simulink model "SdbRxSigProc"  (R2022b) (continued)
== system SdbRxSigProc == (continued)
  SdbRxSigProc_Runnable_sys/64(HmiClimaRow3PwrNgcc_HmiClimaRow3PwrNgcc_write) -> HmiClimaRow3PwrNgcc_HmiClimaRow3PwrNgcc/1
  SdbRxSigProc_Runnable_sys/65(HMIClimaVentSetNgcc_HMIClimaVentSetNgcc_write) -> HMIClimaVentSetNgcc_HMIClimaVentSetNgcc/1
  SdbRxSigProc_Runnable_sys/66(HmiDrvrHeadPosnNgcc_HmiDrvrHeadPosnNgcc_write) -> HmiDrvrHeadPosnNgcc_HmiDrvrHeadPosnNgcc/1
  SdbRxSigProc_Runnable_sys/67(HmiElecAirBodyVentLebtnNgcc_HmiElecAirBodyVentLebtnNgcc_write) -> HmiElecAirBodyVentLebtnNgcc_HmiElecAirBodyVentLebtnNgcc/1
  SdbRxSigProc_Runnable_sys/68(HmiElecAirBodyVentRibtnNgcc_HmiElecAirBodyVentRibtnNgcc_write) -> HmiElecAirBodyVentRibtnNgcc_HmiElecAirBodyVentRibtnNgcc/1
  SdbRxSigProc_Runnable_sys/69(HmiElecAirConsolePosnNgcc_HmiElecAirConsolePosnNgcc_write) -> HmiElecAirConsolePosnNgcc_HmiElecAirConsolePosnNgcc/1
  SdbRxSigProc_Runnable_sys/70(HmiElecAirConsoleSwtLeNgcc_HmiElecAirConsoleSwtLeNgcc_write) -> HmiElecAirConsoleSwtLeNgcc_HmiElecAirConsoleSwtLeNgcc/1
  SdbRxSigProc_Runnable_sys/71(HmiElecAirConsoleSwtRiNgcc_HmiElecAirConsoleSwtRiNgcc_write) -> HmiElecAirConsoleSwtRiNgcc_HmiElecAirConsoleSwtRiNgcc/1
  SdbRxSigProc_Runnable_sys/72(HmiElecAirDirModeSecRowReqNgcc_HmiElecAirDirModeSecRowReqNgcc_write) -> HmiElecAirDirModeSecRowReqNgcc_HmiElecAirDirModeSecRowReqNgcc/1
  SdbRxSigProc_Runnable_sys/73(HmiElecAirDirSwngSecLeNgcc_HmiElecAirDirSwngSecLeNgcc_write) -> HmiElecAirDirSwngSecLeNgcc_HmiElecAirDirSwngSecLeNgcc/1
  SdbRxSigProc_Runnable_sys/74(HmiElecAirDirSwngSecRiNgcc_HmiElecAirDirSwngSecRiNgcc_write) -> HmiElecAirDirSwngSecRiNgcc_HmiElecAirDirSwngSecRiNgcc/1
  SdbRxSigProc_Runnable_sys/75(HmiElecAirDrvrNatureWindReqNgcc_HmiElecAirDrvrNatureWindReqNgcc_write) -> HmiElecAirDrvrNatureWindReqNgcc_HmiElecAirDrvrNatureWindReqNgcc/1
  SdbRxSigProc_Runnable_sys/76(HmiElecAirPassNatureWindReqNgcc_HmiElecAirPassNatureWindReqNgcc_write) -> HmiElecAirPassNatureWindReqNgcc_HmiElecAirPassNatureWindReqNgcc/1
  SdbRxSigProc_Runnable_sys/77(HMIFACSwtNgcc_HMIFACSwtNgcc_write) -> HMIFACSwtNgcc_HMIFACSwtNgcc/1
  SdbRxSigProc_Runnable_sys/78(HmiFanLvlRow1LeNgcc_HmiFanLvlRow1LeNgcc_write) -> HmiFanLvlRow1LeNgcc_HmiFanLvlRow1LeNgcc/1
  SdbRxSigProc_Runnable_sys/79(HmiFanLvlRow1RiNgcc_HmiFanLvlRow1RiNgcc_write) -> HmiFanLvlRow1RiNgcc_HmiFanLvlRow1RiNgcc/1
  SdbRxSigProc_Runnable_sys/80(HmiFragraChRatReq_HmiFragraChRatReq_write) -> HmiFragraChRatReq_HmiFragraChRatReq/1
  SdbRxSigProc_Runnable_sys/81(HmiFragraLvlReq_HmiFragraLvlReq_write) -> HmiFragraLvlReq_HmiFragraLvlReq/1
  SdbRxSigProc_Runnable_sys/82(HMILBRACStrtSetNgcc_HMILBRACStrtSetNgcc_write) -> HMILBRACStrtSetNgcc_HMILBRACStrtSetNgcc/1
  SdbRxSigProc_Runnable_sys/83(HmiOxyinCarSwtNgcc_HmiOxyinCarSwtNgcc_write) -> HmiOxyinCarSwtNgcc_HmiOxyinCarSwtNgcc/1
  SdbRxSigProc_Runnable_sys/84(HmiParkVentSetNgcc_HmiParkVentSetNgcc_write) -> HmiParkVentSetNgcc_HmiParkVentSetNgcc/1
  SdbRxSigProc_Runnable_sys/85(HmiParkVentTmrSetNgcc_HmiParkVentTmrSetNgcc_write) -> HmiParkVentTmrSetNgcc_HmiParkVentTmrSetNgcc/1
  SdbRxSigProc_Runnable_sys/86(HmiPassHeadPosnNgcc_HmiPassHeadPosnNgcc_write) -> HmiPassHeadPosnNgcc_HmiPassHeadPosnNgcc/1
  SdbRxSigProc_Runnable_sys/87(HmiPopUpResp_HmiPopUpResp_write) -> HmiPopUpResp_HmiPopUpResp/1
  SdbRxSigProc_Runnable_sys/88(HmiSmrtWindFolwSetNgcc_HmiSmrtWindFolwSetNgcc_write) -> HmiSmrtWindFolwSetNgcc_HmiSmrtWindFolwSetNgcc/1
  SdbRxSigProc_Runnable_sys/89(HMIThermoTankDoorOpenNgcc_HMIThermoTankDoorOpenNgcc_write) -> HMIThermoTankDoorOpenNgcc_HMIThermoTankDoorOpenNgcc/1
  SdbRxSigProc_Runnable_sys/90(HMIThermoTankEcoSwNgcc_HMIThermoTankEcoSwNgcc_write) -> HMIThermoTankEcoSwNgcc_HMIThermoTankEcoSwNgcc/1
  SdbRxSigProc_Runnable_sys/91(HMIThermoTankTNgcc_HMIThermoTankTNgcc_write) -> HMIThermoTankTNgcc_HMIThermoTankTNgcc/1
  SdbRxSigProc_Runnable_sys/92(HmiThermoTSteDeoNewSwtNgcc_HmiThermoTSteDeoNewSwtNgcc_write) -> HmiThermoTSteDeoNewSwtNgcc_HmiThermoTSteDeoNewSwtNgcc/1
  SdbRxSigProc_Runnable_sys/93(HmiThermoTSteDeoSwtNgcc_HmiThermoTSteDeoSwtNgcc_write) -> HmiThermoTSteDeoSwtNgcc_HmiThermoTSteDeoSwtNgcc/1
  SdbRxSigProc_Runnable_sys/94(HmiUVSterilSetNgcc_HmiUVSterilSetNgcc_write) -> HmiUVSterilSetNgcc_HmiUVSterilSetNgcc/1
  SdbRxSigProc_Runnable_sys/95(HmiWinterLockCarPrevnIceSetNgcc_HmiWinterLockCarPrevnIceSetNgcc_write) -> HmiWinterLockCarPrevnIceSetNgcc_HmiWinterLockCarPrevnIceSetNgcc/1
  SdbRxSigProc_Runnable_sys/96(HoodStsNgcc_HoodStsNgcc_write) -> HoodStsNgcc_HoodStsNgcc/1
  SdbRxSigProc_Runnable_sys/97(HvBattLimnIndcnNgcc_HvBattLimnIndcnNgcc_write) -> HvBattLimnIndcnNgcc_HvBattLimnIndcnNgcc/1
  SdbRxSigProc_Runnable_sys/98(InflateAirSrcSwtNgcc_InflateAirSrcSwtNgcc_write) -> InflateAirSrcSwtNgcc_InflateAirSrcSwtNgcc/1
  SdbRxSigProc_Runnable_sys/99(InpVoltFbNgcc_InpVoltFbNgcc_write) -> InpVoltFbNgcc_InpVoltFbNgcc/1
  SdbRxSigProc_Runnable_sys/100(LiOprnModNgcc_LiOprnModNgcc_write) -> LiOprnModNgcc_LiOprnModNgcc/1
  SdbRxSigProc_Runnable_sys/101(LonglifeBatteryReqNgcc_LonglifeBatteryReqNgcc_write) -> LonglifeBatteryReqNgcc_LonglifeBatteryReqNgcc/1
  SdbRxSigProc_Runnable_sys/102(McuOvrTempErrNgcc_McuOvrTempErrNgcc_write) -> McuOvrTempErrNgcc_McuOvrTempErrNgcc/1
  SdbRxSigProc_Runnable_sys/103(McuTempFbNgcc_McuTempFbNgcc_write) -> McuTempFbNgcc_McuTempFbNgcc/1
  SdbRxSigProc_Runnable_sys/104(McuTempSnsrErrNgcc_McuTempSnsrErrNgcc_write) -> McuTempSnsrErrNgcc_McuTempSnsrErrNgcc/1
  SdbRxSigProc_Runnable_sys/105(MoleculeVlv1Ngcc_MoleculeVlv1Ngcc_write) -> MoleculeVlv1Ngcc_MoleculeVlv1Ngcc/1
  SdbRxSigProc_Runnable_sys/106(MoleculeVlv2Ngcc_MoleculeVlv2Ngcc_write) -> MoleculeVlv2Ngcc_MoleculeVlv2Ngcc/1
  SdbRxSigProc_Runnable_sys/107(MosOvrTempErrNgcc_MosOvrTempErrNgcc_write) -> MosOvrTempErrNgcc_MosOvrTempErrNgcc/1
  SdbRxSigProc_Runnable_sys/108(MosTempFbNgcc_MosTempFbNgcc_write) -> MosTempFbNgcc_MosTempFbNgcc/1
  SdbRxSigProc_Runnable_sys/109(MosTempSnsrErrNgcc_MosTempSnsrErrNgcc_write) -> MosTempSnsrErrNgcc_MosTempSnsrErrNgcc/1
  SdbRxSigProc_Runnable_sys/110(MotStsFrmOGMNgcc_MotStsFrmOGMNgcc_write) -> MotStsFrmOGMNgcc_MotStsFrmOGMNgcc/1
  SdbRxSigProc_Runnable_sys/111(MotTempFbNgcc_MotTempFbNgcc_write) -> MotTempFbNgcc_MotTempFbNgcc/1
  SdbRxSigProc_Runnable_sys/112(MotTempSnsrErrNgcc_MotTempSnsrErrNgcc_write) -> MotTempSnsrErrNgcc_MotTempSnsrErrNgcc/1
  SdbRxSigProc_Runnable_sys/113(OGMCmprSpdFbNgcc_OGMCmprSpdFbNgcc_write) -> OGMCmprSpdFbNgcc_OGMCmprSpdFbNgcc/1
  SdbRxSigProc_Runnable_sys/114(OGMFlowFbNgcc_OGMFlowFbNgcc_write) -> OGMFlowFbNgcc_OGMFlowFbNgcc/1
  SdbRxSigProc_Runnable_sys/115(OGMHwVersNgcc_OGMHwVersNgcc_write) -> OGMHwVersNgcc_OGMHwVersNgcc/1
  SdbRxSigProc_Runnable_sys/116(OGMIntErrNgcc_OGMIntErrNgcc_write) -> OGMIntErrNgcc_OGMIntErrNgcc/1
  SdbRxSigProc_Runnable_sys/117(OGMOvrVoltRangErrNgcc_OGMOvrVoltRangErrNgcc_write) -> OGMOvrVoltRangErrNgcc_OGMOvrVoltRangErrNgcc/1
  SdbRxSigProc_Runnable_sys/118(OGMRunTiNgcc_OGMRunTiNgcc_write) -> OGMRunTiNgcc_OGMRunTiNgcc/1
  SdbRxSigProc_Runnable_sys/119(OGMSwVersNgcc_OGMSwVersNgcc_write) -> OGMSwVersNgcc_OGMSwVersNgcc/1
  SdbRxSigProc_Runnable_sys/120(OGMTempErrNgcc_OGMTempErrNgcc_write) -> OGMTempErrNgcc_OGMTempErrNgcc/1
  SdbRxSigProc_Runnable_sys/121(OGMUndVoltRangErrNgcc_OGMUndVoltRangErrNgcc_write) -> OGMUndVoltRangErrNgcc_OGMUndVoltRangErrNgcc/1
  SdbRxSigProc_Runnable_sys/122(OxygenConcFbFrmOGMNgcc_OxygenConcFbFrmOGMNgcc_write) -> OxygenConcFbFrmOGMNgcc_OxygenConcFbFrmOGMNgcc/1
  SdbRxSigProc_Runnable_sys/123(OxygenConcFltNgcc_OxygenConcFltNgcc_write) -> OxygenConcFltNgcc_OxygenConcFltNgcc/1
  SdbRxSigProc_Runnable_sys/124(OxygenConcSnsrElecErrNgcc_OxygenConcSnsrElecErrNgcc_write) -> OxygenConcSnsrElecErrNgcc_OxygenConcSnsrElecErrNgcc/1
  SdbRxSigProc_Runnable_sys/125(OxygenConcSnsrIntErrNgcc_OxygenConcSnsrIntErrNgcc_write) -> OxygenConcSnsrIntErrNgcc_OxygenConcSnsrIntErrNgcc/1
  SdbRxSigProc_Runnable_sys/126(OxygenConcSnsrOvrVoltRangErrNgcc_OxygenConcSnsrOvrVoltRangErrNgcc_write) -> OxygenConcSnsrOvrVoltRangErrNgcc_OxygenConcSnsrOvrVoltRangErrNgcc/1
  SdbRxSigProc_Runnable_sys/127(OxygenConcSnsrStsFrmOGMNgcc_OxygenConcSnsrStsFrmOGMNgcc_write) -> OxygenConcSnsrStsFrmOGMNgcc_OxygenConcSnsrStsFrmOGMNgcc/1
  SdbRxSigProc_Runnable_sys/128(OxygenConcSnsrStsNgcc_OxygenConcSnsrStsNgcc_write) -> OxygenConcSnsrStsNgcc_OxygenConcSnsrStsNgcc/1
  SdbRxSigProc_Runnable_sys/129(OxygenConcSnsrTempErrNgcc_OxygenConcSnsrTempErrNgcc_write) -> OxygenConcSnsrTempErrNgcc_OxygenConcSnsrTempErrNgcc/1
  SdbRxSigProc_Runnable_sys/130(OxygenConcSnsrUndVoltRangErrNgcc_OxygenConcSnsrUndVoltRangErrNgcc_write) -> OxygenConcSnsrUndVoltRangErrNgcc_OxygenConcSnsrUndVoltRangErrNgcc/1
  SdbRxSigProc_Runnable_sys/131(OxygenGenrErrInfoNgcc_OxygenGenrErrInfoNgcc_write) -> OxygenGenrErrInfoNgcc_OxygenGenrErrInfoNgcc/1
  SdbRxSigProc_Runnable_sys/132(OxygenSplyAvlStsNgcc_OxygenSplyAvlStsNgcc_write) -> OxygenSplyAvlStsNgcc_OxygenSplyAvlStsNgcc/1
  SdbRxSigProc_Runnable_sys/133(OxygenSplyErrNgcc_OxygenSplyErrNgcc_write) -> OxygenSplyErrNgcc_OxygenSplyErrNgcc/1
  SdbRxSigProc_Runnable_sys/134(OxygenTubeBlkNgcc_OxygenTubeBlkNgcc_write) -> OxygenTubeBlkNgcc_OxygenTubeBlkNgcc/1
  SdbRxSigProc_Runnable_sys/135(PassSeatPosPercNgcc_PassSeatPosPercNgcc_write) -> PassSeatPosPercNgcc_PassSeatPosPercNgcc/1
  SdbRxSigProc_Runnable_sys/136(PassSeatSts2_Ngcc_PassSeatSts2_write) -> PassSeatSts2_Ngcc_PassSeatSts2/1
  SdbRxSigProc_Runnable_sys/137(PassSeatVentnLvlSts2Ngcc_PassSeatVentnLvlSts2Ngcc_write) -> PassSeatVentnLvlSts2Ngcc_PassSeatVentnLvlSts2Ngcc/1
  SdbRxSigProc_Runnable_sys/138(PhaseCurrentFbNgcc_PhaseCurrentFbNgcc_write) -> PhaseCurrentFbNgcc_PhaseCurrentFbNgcc/1
  SdbRxSigProc_Runnable_sys/139(PosnBriefNgcc_PosnBriefNgcc_write) -> PosnBriefNgcc_PosnBriefNgcc/1
  SdbRxSigProc_Runnable_sys/140(PressureFbNgcc_PressureFbNgcc_write) -> PressureFbNgcc_PressureFbNgcc/1
  SdbRxSigProc_Runnable_sys/141(PressureFltNgcc_PressureFltNgcc_write) -> PressureFltNgcc_PressureFltNgcc/1
  SdbRxSigProc_Runnable_sys/142(PressureSnsrErrNgcc_PressureSnsrErrNgcc_write) -> PressureSnsrErrNgcc_PressureSnsrErrNgcc/1
  SdbRxSigProc_Runnable_sys/143(PwrCnsForHvacHeatrElecRe_PwrCnsForHvacHeatrElecRe_write) -> PwrCnsForHvacHeatrElecRe_PwrCnsForHvacHeatrElecRe/1
  SdbRxSigProc_Runnable_sys/144(RefrLpSwStsNgcc_RefrLpSwStsNgcc_write) -> RefrLpSwStsNgcc_RefrLpSwStsNgcc/1
  SdbRxSigProc_Runnable_sys/145(RefrtorVlvEnaNgcc_RefrtorVlvEnaNgcc_write) -> RefrtorVlvEnaNgcc_RefrtorVlvEnaNgcc/1
  SdbRxSigProc_Runnable_sys/146(RoadInclnNgcc_RoadInclnNgcc_write) -> RoadInclnNgcc_RoadInclnNgcc/1
  SdbRxSigProc_Runnable_sys/147(RuranACModeSetNgcc_RuranACModeSetNgcc_write) -> RuranACModeSetNgcc_RuranACModeSetNgcc/1
  SdbRxSigProc_Runnable_sys/148(SceneModSeld_Ngcc_SceneModSeld_write) -> SceneModSeld_Ngcc_SceneModSeld/1
  SdbRxSigProc_Runnable_sys/149(SeatBackAngleRowFirstDrvrNgcc_SeatBackAngleRowFirstDrvrNgcc_write) -> SeatBackAngleRowFirstDrvrNgcc_SeatBackAngleRowFirstDrvrNgcc/1
  SdbRxSigProc_Runnable_sys/150(SeatBackAngleRowFirstPassNgcc_SeatBackAngleRowFirstPassNgcc_write) -> SeatBackAngleRowFirstPassNgcc_SeatBackAngleRowFirstPassNgcc/1
  SdbRxSigProc_Runnable_sys/151(SeatOccptAtRowSecDrSide2_Ngcc_SeatOccptAtRowSecDrSide2_write) -> SeatOccptAtRowSecDrSide2_Ngcc_SeatOccptAtRowSecDrSide2/1
  SdbRxSigProc_Runnable_sys/152(SeatOccptAtRowSecMid2_Ngcc_SeatOccptAtRowSecMid2_write) -> SeatOccptAtRowSecMid2_Ngcc_SeatOccptAtRowSecMid2/1
  SdbRxSigProc_Runnable_sys/153(SeatOccptAtRowSecPasSide2_Ngcc_SeatOccptAtRowSecPasSide2_write) -> SeatOccptAtRowSecPasSide2_Ngcc_SeatOccptAtRowSecPasSide2/1
  SdbRxSigProc_Runnable_sys/154(SeatOccptAtRowThrdLe2Ngcc_SeatOccptAtRowThrdLe2Ngcc_write) -> SeatOccptAtRowThrdLe2Ngcc_SeatOccptAtRowThrdLe2Ngcc/1
  SdbRxSigProc_Runnable_sys/155(SeatOccptAtRowThrdRi2Ngcc_SeatOccptAtRowThrdRi2Ngcc_write) -> SeatOccptAtRowThrdRi2Ngcc_SeatOccptAtRowThrdRi2Ngcc/1
  SdbRxSigProc_Runnable_sys/156(SportModeThmgtRequestNgcc_SportModeThmgtRequestNgcc_write) -> SportModeThmgtRequestNgcc_SportModeThmgtRequestNgcc/1
  SdbRxSigProc_Runnable_sys/157(TCSCooltFlowNgcc_TCSCooltFlowNgcc_write) -> TCSCooltFlowNgcc_TCSCooltFlowNgcc/1
  SdbRxSigProc_Runnable_sys/158(TCSCooltTNgcc_TCSCooltTNgcc_write) -> TCSCooltTNgcc_TCSCooltTNgcc/1
  SdbRxSigProc_Runnable_sys/159(TcsDoorEleBailReqNgcc_TcsDoorEleBailReqNgcc_write) -> TcsDoorEleBailReqNgcc_TcsDoorEleBailReqNgcc/1
  SdbRxSigProc_Runnable_sys/160(TcsFanReqNgcc_TcsFanReqNgcc_write) -> TcsFanReqNgcc_TcsFanReqNgcc/1
  SdbRxSigProc_Runnable_sys/161(TcsModSwtReqNgcc_TcsModSwtReqNgcc_write) -> TcsModSwtReqNgcc_TcsModSwtReqNgcc/1
  SdbRxSigProc_Runnable_sys/162(TcsOvrheatdProtnStsNgcc_TcsOvrheatdProtnStsNgcc_write) -> TcsOvrheatdProtnStsNgcc_TcsOvrheatdProtnStsNgcc/1
  SdbRxSigProc_Runnable_sys/163(TcsSemiconReqNgcc_TcsSemiconReqNgcc_write) -> TcsSemiconReqNgcc_TcsSemiconReqNgcc/1
  SdbRxSigProc_Runnable_sys/164(TcsTmrOverReqNgcc_TcsTmrOverReqNgcc_write) -> TcsTmrOverReqNgcc_TcsTmrOverReqNgcc/1
  SdbRxSigProc_Runnable_sys/165(TcsTNowCtrlReqNgcc_TcsTNowCtrlReqNgcc_write) -> TcsTNowCtrlReqNgcc_TcsTNowCtrlReqNgcc/1
  SdbRxSigProc_Runnable_sys/166(TcsTopDoorEleBailReqNgcc_TcsTopDoorEleBailReqNgcc_write) -> TcsTopDoorEleBailReqNgcc_TcsTopDoorEleBailReqNgcc/1
  SdbRxSigProc_Runnable_sys/167(TelmOxygenValReqNgcc_TelmOxygenValReqNgcc_write) -> TelmOxygenValReqNgcc_TelmOxygenValReqNgcc/1
  SdbRxSigProc_Runnable_sys/168(TelmThermoTankECOSetNgcc_TelmThermoTankECOSetNgcc_write) -> TelmThermoTankECOSetNgcc_TelmThermoTankECOSetNgcc/1
  SdbRxSigProc_Runnable_sys/169(TelmThermoTankTNgcc_TelmThermoTankTNgcc_write) -> TelmThermoTankTNgcc_TelmThermoTankTNgcc/1
  SdbRxSigProc_Runnable_sys/170(ThermHvacRecircReqEnaNgcc_ThermHvacRecircReqEnaNgcc_write) -> ThermHvacRecircReqEnaNgcc_ThermHvacRecircReqEnaNgcc/1
  SdbRxSigProc_Runnable_sys/171(ThermHvacRecircReqNgcc_ThermHvacRecircReqNgcc_write) -> ThermHvacRecircReqNgcc_ThermHvacRecircReqNgcc/1
  SdbRxSigProc_Runnable_sys/172(ThrdRowSeatHeatgActlPwrCnsLeNgcc_ThrdRowSeatHeatgActlPwrCnsLeNgcc_write) -> ThrdRowSeatHeatgActlPwrCnsLeNgcc_ThrdRowSeatHeatgActlPwrCnsLeNgcc/1
  SdbRxSigProc_Runnable_sys/173(ThrdRowSeatHeatgActlPwrCnsRiNgcc_ThrdRowSeatHeatgActlPwrCnsRiNgcc_write) -> ThrdRowSeatHeatgActlPwrCnsRiNgcc_ThrdRowSeatHeatgActlPwrCnsRiNgcc/1
  SdbRxSigProc_Runnable_sys/174(ThrdRowSeatHeatgDesPwrCnsLeNgcc_ThrdRowSeatHeatgDesPwrCnsLeNgcc_write) -> ThrdRowSeatHeatgDesPwrCnsLeNgcc_ThrdRowSeatHeatgDesPwrCnsLeNgcc/1
  SdbRxSigProc_Runnable_sys/175(ThrdRowSeatHeatgDesPwrCnsRiNgcc_ThrdRowSeatHeatgDesPwrCnsRiNgcc_write) -> ThrdRowSeatHeatgDesPwrCnsRiNgcc_ThrdRowSeatHeatgDesPwrCnsRiNgcc/1
  SdbRxSigProc_Runnable_sys/176(ThrdRowSeatVentnActlPwrCnsLeNgcc_ThrdRowSeatVentnActlPwrCnsLeNgcc_write) -> ThrdRowSeatVentnActlPwrCnsLeNgcc_ThrdRowSeatVentnActlPwrCnsLeNgcc/1
  SdbRxSigProc_Runnable_sys/177(ThrdRowSeatVentnActlPwrCnsRiNgcc_ThrdRowSeatVentnActlPwrCnsRiNgcc_write) -> ThrdRowSeatVentnActlPwrCnsRiNgcc_ThrdRowSeatVentnActlPwrCnsRiNgcc/1
  SdbRxSigProc_Runnable_sys/178(ThrdRowSeatVentnDesPwrCnsLeNgcc_ThrdRowSeatVentnDesPwrCnsLeNgcc_write) -> ThrdRowSeatVentnDesPwrCnsLeNgcc_ThrdRowSeatVentnDesPwrCnsLeNgcc/1
  SdbRxSigProc_Runnable_sys/179(ThrdRowSeatVentnDesPwrCnsRiNgcc_ThrdRowSeatVentnDesPwrCnsRiNgcc_write) -> ThrdRowSeatVentnDesPwrCnsRiNgcc_ThrdRowSeatVentnDesPwrCnsRiNgcc/1
  SdbRxSigProc_Runnable_sys/180(UseEvapReqTMinNewNgcc_UseEvapReqTMinNewNgcc_write) -> UseEvapReqTMinNewNgcc_UseEvapReqTMinNewNgcc/1
  SdbRxSigProc_Runnable_sys/181(VehTiAndDataNgcc_VehTiAndDataNgcc_write) -> VehTiAndDataNgcc_VehTiAndDataNgcc/1
  SdbRxSigProc_Runnable_sys/182(VlvVoltFltNgcc_VlvVoltFltNgcc_write) -> VlvVoltFltNgcc_VlvVoltFltNgcc/1
  SdbRxSigProc_Runnable_sys/183(WarnLvlMsgNgcc_WarnLvlMsgNgcc_write) -> WarnLvlMsgNgcc_WarnLvlMsgNgcc/1
  SdbRxSigProc_Runnable_sys/184(WasRespLevelNgcc_WasRespLevelNgcc_write) -> WasRespLevelNgcc_WasRespLevelNgcc/1
  SdbRxSigProc_Runnable_sys/185(WaterDepthInformationNgcc_WaterDepthInformationNgcc_write) -> WaterDepthInformationNgcc_WaterDepthInformationNgcc/1
  SdbRxSigProc_Runnable_sys/186(WeatherQualityAQINgcc_WeatherQualityAQINgcc_write) -> WeatherQualityAQINgcc_WeatherQualityAQINgcc/1
  SdbRxSigProc_Runnable_sys/187(WinPosnStsDrv_WinPosnStsAtDrv_write) -> WinPosnStsDrv_WinPosnStsAtDrv/1
  SdbRxSigProc_Runnable_sys/188(ActvnOfWshrFrntSafe_Ngcc_ActvnOfWshrFrntSafe_write) -> ActvnOfWshrFrntSafe_Ngcc_ActvnOfWshrFrntSafe/1
  SdbRxSigProc_Runnable_sys/189(AirMtrlSnsrInAirQlyIdx_AirMtrlSnsrInAirQlyIdx_write) -> AirMtrlSnsrInAirQlyIdx_AirMtrlSnsrInAirQlyIdx/1
  SdbRxSigProc_Runnable_sys/190(AirTAftHeatElmEstimd_AirTAftHeatElmEstimd_write) -> AirTAftHeatElmEstimd_AirTAftHeatElmEstimd/1
  SdbRxSigProc_Runnable_sys/191(AirTFromHeatgEstimd_Ngcc_AirTFromHeatgEstimd_write) -> AirTFromHeatgEstimd_Ngcc_AirTFromHeatgEstimd/1
  AmbTOutOfVeh_AmbTOutOfVeh_merge/1 -> AmbTOutOfVeh_AmbTOutOfVeh/1
  AmbTRaw_Ngcc_AmbTRaw_merge/1 -> AmbTRaw_Ngcc_AmbTRaw/1
  SdbRxSigProc_Runnable_sys/194(AsyFanSts_AsyFanSts_write) -> AsyFanSts_AsyFanSts/1
  SdbRxSigProc_Runnable_sys/195(BltLockStAtDrvr_Ngcc_BltLockStAtDrvr_write) -> BltLockStAtDrvr_Ngcc_BltLockStAtDrvr/1
  SdbRxSigProc_Runnable_sys/196(BltLockStAtPass_Ngcc_BltLockStAtPass_write) -> BltLockStAtPass_Ngcc_BltLockStAtPass/1
  SdbRxSigProc_Runnable_sys/197(BltLockStAtRowSecLe_Ngcc_BltLockStAtRowSecLe_write) -> BltLockStAtRowSecLe_Ngcc_BltLockStAtRowSecLe/1
  SdbRxSigProc_Runnable_sys/198(BltLockStAtRowSecMid_Ngcc_BltLockStAtRowSecMid_write) -> BltLockStAtRowSecMid_Ngcc_BltLockStAtRowSecMid/1
  SdbRxSigProc_Runnable_sys/199(BltLockStAtRowSecRi_Ngcc_BltLockStAtRowSecRi_write) -> BltLockStAtRowSecRi_Ngcc_BltLockStAtRowSecRi/1
  SdbRxSigProc_Runnable_sys/200(BltLockStAtRowThrdLe_Ngcc_BltLockStAtRowThrdLe_write) -> BltLockStAtRowThrdLe_Ngcc_BltLockStAtRowThrdLe/1
  SdbRxSigProc_Runnable_sys/201(BltLockStAtRowThrdRi_Ngcc_BltLockStAtRowThrdRi_write) -> BltLockStAtRowThrdRi_Ngcc_BltLockStAtRowThrdRi/1
  SdbRxSigProc_Runnable_sys/202(CamHeatrActv_CamHeatrActv_write) -> CamHeatrActv_CamHeatrActv/1
  SdbRxSigProc_Runnable_sys/203(CarTiGlb_Ngcc_CarTiGlb_write) -> CarTiGlb_Ngcc_CarTiGlb/1
  SdbRxSigProc_Runnable_sys/204(ClimaCmd_Ngcc_ClimaCmd_write) -> ClimaCmd_Ngcc_ClimaCmd/1
  SdbRxSigProc_Runnable_sys/205(ClimaPwrAllwd_Ngcc_ClimaPwrAllwd_write) -> ClimaPwrAllwd_Ngcc_ClimaPwrAllwd/1
  SdbRxSigProc_Runnable_sys/206(ClimaRqrd1ConnGen2_ClimaRqrd1ConnGen2_write) -> ClimaRqrd1ConnGen2_ClimaRqrd1ConnGen2/1
  SdbRxSigProc_Runnable_sys/207(ClimaRqrdFromRem1_ClimaRqrdFromRem1_write) -> ClimaRqrdFromRem1_ClimaRqrdFromRem1/1
  SdbRxSigProc_Runnable_sys/208(ClimaTmr_ClimaTmr_write) -> ClimaTmr_ClimaTmr/1
  SdbRxSigProc_Runnable_sys/209(ClimaTmrStsRqrd_ClimaTmrStsRqrd_write) -> ClimaTmrStsRqrd_ClimaTmrStsRqrd/1
  SdbRxSigProc_Runnable_sys/210(ClngRqrdFromHmi_ClngRqrdFromHmi_write) -> ClngRqrdFromHmi_ClngRqrdFromHmi/1
  SdbRxSigProc_Runnable_sys/211(ClngRqrdFromTelm_ClngRqrdFromTelm_write) -> ClngRqrdFromTelm_ClngRqrdFromTelm/1
  SdbRxSigProc_Runnable_sys/212(ClngRqrdFromTelmConnGen2_ClngRqrdFromTelmConnGen2_write) -> ClngRqrdFromTelmConnGen2_ClngRqrdFromTelmConnGen2/1
  SdbRxSigProc_Runnable_sys/213(CmpmtInAirPmAQI_Ngcc_CmpmtInAirPmAQI_write) -> CmpmtInAirPmAQI_Ngcc_CmpmtInAirPmAQI/1
  SdbRxSigProc_Runnable_sys/214(CmpmtInAirPmDnsty_Ngcc_CmpmtInAirPmDnsty_write) -> CmpmtInAirPmDnsty_Ngcc_CmpmtInAirPmDnsty/1
  SdbRxSigProc_Runnable_sys/215(CmptmtClngPreStrtReqNgcc_CmptmtClngPreStrtReq_write) -> CmptmtClngPreStrtReqNgcc_CmptmtClngPreStrtReq/1
  SdbRxSigProc_Runnable_sys/216(CmptmtCoolgSts_Ngcc_CmptmtCoolgSts_write) -> CmptmtCoolgSts_Ngcc_CmptmtCoolgSts/1
  CmptmtRelHum_Ngcc_CmptmtRelHum_merge/1 -> CmptmtRelHum_Ngcc_CmptmtRelHum/1
  SdbRxSigProc_Runnable_sys/218(CoolgLoad_CoolgLoad_write) -> CoolgLoad_CoolgLoad/1
  SdbRxSigProc_Runnable_sys/219(CoolgReqForDispCen_CoolgReqForDispCen_write) -> CoolgReqForDispCen_CoolgReqForDispCen/1
  SdbRxSigProc_Runnable_sys/220(CoolgReqForDrvrDisp_CoolgReqForDrvrDisp_write) -> CoolgReqForDrvrDisp_CoolgReqForDrvrDisp/1
  CooltHeatrAvlStsForPrkgClima_CooltHeatrAvlStsForPrkgClima_merge/1 -> CooltHeatrAvlStsForPrkgClima_CooltHeatrAvlStsForPrkgClima/1
  SdbRxSigProc_Runnable_sys/222(CooltTForPrkgClima_CooltTForPrkgClima_write) -> CooltTForPrkgClima_CooltTForPrkgClima/1
  SdbRxSigProc_Runnable_sys/223(CooltTInHeatElm_CooltTInHeatElm_write) -> CooltTInHeatElm_CooltTInHeatElm/1
  SdbRxSigProc_Runnable_sys/224(CooltVlvClsd_CooltVlvClsd_write) -> CooltVlvClsd_CooltVlvClsd/1
  SdbRxSigProc_Runnable_sys/225(CupHoldrDesPwr_CupHoldrDesPwr_write) -> CupHoldrDesPwr_CupHoldrDesPwr/1
  SdbRxSigProc_Runnable_sys/226(DefrstSts_Ngcc_DefrstSts_write) -> DefrstSts_Ngcc_DefrstSts/1
  SdbRxSigProc_Runnable_sys/227(DoorDrvrReSts_DoorDrvrReSts_write) -> DoorDrvrReSts_DoorDrvrReSts/1
  SdbRxSigProc_Runnable_sys/228(DoorDrvrSts_Ngcc_DoorDrvrSts_write) -> DoorDrvrSts_Ngcc_DoorDrvrSts/1
  SdbRxSigProc_Runnable_sys/229(DoorPassReSts_DoorPassReSts_write) -> DoorPassReSts_DoorPassReSts/1
  SdbRxSigProc_Runnable_sys/230(DoorPassSts_Ngcc_DoorPassSts_write) -> DoorPassSts_Ngcc_DoorPassSts/1
  SdbRxSigProc_Runnable_sys/231(DrvModReq_Ngcc_DrvModReq_write) -> DrvModReq_Ngcc_DrvModReq/1
  SdbRxSigProc_Runnable_sys/232(DrvrAirCdnrSetg_Ngcc_DrvrAirCdnrSetg_write) -> DrvrAirCdnrSetg_Ngcc_DrvrAirCdnrSetg/1
  SdbRxSigProc_Runnable_sys/233(DrvrSeatHeatgActlPwrCns_Ngcc_DrvrSeatHeatgActlPwrCns_write) -> DrvrSeatHeatgActlPwrCns_Ngcc_DrvrSeatHeatgActlPwrCns/1
  SdbRxSigProc_Runnable_sys/234(DrvrSeatHeatgDesPwrCns_Ngcc_DrvrSeatHeatgDesPwrCns_write) -> DrvrSeatHeatgDesPwrCns_Ngcc_DrvrSeatHeatgDesPwrCns/1
  SdbRxSigProc_Runnable_sys/235(DrvrSeatVentnDesPwrCns_Ngcc_DrvrSeatVentnDesPwrCns_write) -> DrvrSeatVentnDesPwrCns_Ngcc_DrvrSeatVentnDesPwrCns/1
  SdbRxSigProc_Runnable_sys/236(EcuKeepActvFromHeatg_EcuKeepActvFromHeatg_write) -> EcuKeepActvFromHeatg_EcuKeepActvFromHeatg/1
  SdbRxSigProc_Runnable_sys/237(EgyCnsAllwdForClima_Ngcc_EgyCnsAllwdForClima_write) -> EgyCnsAllwdForClima_Ngcc_EgyCnsAllwdForClima/1
  SdbRxSigProc_Runnable_sys/238(EgySave_EgySave_write) -> EgySave_EgySave/1
  SdbRxSigProc_Runnable_sys/239(EngReqInHybForHeatg_EngReqInHybForHeatg_write) -> EngReqInHybForHeatg_EngReqInHybForHeatg/1
  SdbRxSigProc_Runnable_sys/240(EngSt1WdSts_Ngcc_EngSt1WdSts_write) -> EngSt1WdSts_Ngcc_EngSt1WdSts/1
  EngT_Ngcc_EngT_merge/1 -> EngT_Ngcc_EngT/1
  FogOutOfVeh_FogOutOfVeh_merge/1 -> FogOutOfVeh_FogOutOfVeh/1
  SdbRxSigProc_Runnable_sys/243(HeatdCupPwrCnsAct_HeatdCupPwrCnsAct_write) -> HeatdCupPwrCnsAct_HeatdCupPwrCnsAct/1
  SdbRxSigProc_Runnable_sys/244(HeatgPwrDmdEstimd_HeatgPwrDmdEstimd_write) -> HeatgPwrDmdEstimd_HeatgPwrDmdEstimd/1
  SdbRxSigProc_Runnable_sys/245(HeatrActvnReq_HeatrActvnReq_write) -> HeatrActvnReq_HeatrActvnReq/1
  SdbRxSigProc_Runnable_sys/246(HMIClimaEgySaveReq_Ngcc_HMIClimaEgySaveReq_write) -> HMIClimaEgySaveReq_Ngcc_HMIClimaEgySaveReq/1
  SdbRxSigProc_Runnable_sys/247(HmiClimaFrntAutReq_HmiClimaFrntAutReq_write) -> HmiClimaFrntAutReq_HmiClimaFrntAutReq/1
  SdbRxSigProc_Runnable_sys/248(HmiClimaReAutReq_HmiClimaReAutReq_write) -> HmiClimaReAutReq_HmiClimaReAutReq/1
  SdbRxSigProc_Runnable_sys/249(HmiCmptmtAirDistbnFrntLe_HmiCmptmtAirDistbnFrntLe_write) -> HmiCmptmtAirDistbnFrntLe_HmiCmptmtAirDistbnFrntLe/1
  SdbRxSigProc_Runnable_sys/250(HmiCmptmtAirDistbnRe_HmiCmptmtAirDistbnRe_write) -> HmiCmptmtAirDistbnRe_HmiCmptmtAirDistbnRe/1
  SdbRxSigProc_Runnable_sys/251(HmiCmptmtCoolgReq_HmiCmptmtCoolgReq_write) -> HmiCmptmtCoolgReq_HmiCmptmtCoolgReq/1
  SdbRxSigProc_Runnable_sys/252(HmiDefrstMaxReq_HmiDefrstMaxReq_write) -> HmiDefrstMaxReq_HmiDefrstMaxReq/1
  SdbRxSigProc_Runnable_sys/253(HmiHvacFanLvlFrnt_HmiHvacFanLvlFrnt_write) -> HmiHvacFanLvlFrnt_HmiHvacFanLvlFrnt/1
  SdbRxSigProc_Runnable_sys/254(HmiHvacFanLvlRe_HmiHvacFanLvlRe_write) -> HmiHvacFanLvlRe_HmiHvacFanLvlRe/1
  SdbRxSigProc_Runnable_sys/255(HmiMaxACReq_HmiMaxACReq_write) -> HmiMaxACReq_HmiMaxACReq/1
  SdbRxSigProc_Runnable_sys/256(HmiPassTempSyncSet_HmiPassTempSyncSet_write) -> HmiPassTempSyncSet_HmiPassTempSyncSet/1
  SdbRxSigProc_Runnable_sys/257(HpcDeiceReq_Ngcc_HpcDeiceReq_write) -> HpcDeiceReq_Ngcc_HpcDeiceReq/1
  HumOutOfVeh_HumOutOfVeh_merge/1 -> HumOutOfVeh_HumOutOfVeh/1
  SdbRxSigProc_Runnable_sys/259(HvacFanIFrnt_HvacFanIFrnt_write) -> HvacFanIFrnt_HvacFanIFrnt/1
  SdbRxSigProc_Runnable_sys/260(HvacFanIRe_HvacFanIRe_write) -> HvacFanIRe_HvacFanIRe/1
  SdbRxSigProc_Runnable_sys/261(HvacFanMotURe_HvacFanMotURe_write) -> HvacFanMotURe_HvacFanMotURe/1
  SdbRxSigProc_Runnable_sys/262(HvacFanSpdFrnt_HvacFanSpdFrnt_write) -> HvacFanSpdFrnt_HvacFanSpdFrnt/1
  HvBattCoolgPwrHeatgMgrThermPred_HvBattCoolgPwrHeatgMgrThermPred_merge/1 -> HvBattCoolgPwrHeatgMgrThermPred_HvBattCoolgPwrHeatgMgrThermPred/1
  SdbRxSigProc_Runnable_sys/264(HvPwrEgyPrio_Ngcc_HvPwrEgyPrio_write) -> HvPwrEgyPrio_Ngcc_HvPwrEgyPrio/1
  SdbRxSigProc_Runnable_sys/265(IHUAPSts_Ngcc_IHUAPSts_write) -> IHUAPSts_Ngcc_IHUAPSts/1
  SdbRxSigProc_Runnable_sys/266(IncomingAirQlyCtrlFromHmi_Ngcc_IncomingAirQlyCtrlFromHmi_write) -> IncomingAirQlyCtrlFromHmi_Ngcc_IncomingAirQlyCtrlFromHmi/1
  SdbRxSigProc_Runnable_sys/267(IncomingAirQlyPopUpReq_Ngcc_IncomingAirQlyPopUpReq_write) -> IncomingAirQlyPopUpReq_Ngcc_IncomingAirQlyPopUpReq/1
  SdbRxSigProc_Runnable_sys/268(InPm25FanErr_Ngcc_InPm25FanErr_write) -> InPm25FanErr_Ngcc_InPm25FanErr/1
  SdbRxSigProc_Runnable_sys/269(InPm25SnsrSts_Ngcc_InPm25SnsrSts_write) -> InPm25SnsrSts_Ngcc_InPm25SnsrSts/1
  SdbRxSigProc_Runnable_sys/270(InteCleanUnpleSmell_Ngcc_InteCleanUnpleSmell_write) -> InteCleanUnpleSmell_Ngcc_InteCleanUnpleSmell/1
  IsgMotTInt_IsgMotTInt_merge/1 -> IsgMotTInt_IsgMotTInt/1
  SdbRxSigProc_Runnable_sys/272(LoadForCmprForAirCdn_LoadForCmprForAirCdn_write) -> LoadForCmprForAirCdn_LoadForCmprForAirCdn/1
  SdbRxSigProc_Runnable_sys/273(LockgCenSts_Ngcc_LockgCenSts_write) -> LockgCenSts_Ngcc_LockgCenSts/1
  SdbRxSigProc_Runnable_sys/274(MemSaveFlgForCooltHeatrActvnCtrl_MemSaveFlgForCooltHeatrActvnCtrl_write) -> MemSaveFlgForCooltHeatrActvnCtrl_MemSaveFlgForCooltHeatrActvnCtrl/1
  SdbRxSigProc_Runnable_sys/275(MemSaveFlgForCooltHeatrCtrl_MemSaveFlgForCooltHeatrCtrl_write) -> MemSaveFlgForCooltHeatrCtrl_MemSaveFlgForCooltHeatrCtrl/1
  SdbRxSigProc_Runnable_sys/276(MemSaveFlgForFuTInHeatrMdl_MemSaveFlgForFuTInHeatrMdl_write) -> MemSaveFlgForFuTInHeatrMdl_MemSaveFlgForFuTInHeatrMdl/1
  SdbRxSigProc_Runnable_sys/277(MemSaveFlgForHeatgInpMgr_MemSaveFlgForHeatgInpMgr_write) -> MemSaveFlgForHeatgInpMgr_MemSaveFlgForHeatgInpMgr/1
  SdbRxSigProc_Runnable_sys/278(MemSaveFlgForSnsrMdlCooltT_MemSaveFlgForSnsrMdlCooltT_write) -> MemSaveFlgForSnsrMdlCooltT_MemSaveFlgForSnsrMdlCooltT/1
  MemWrForCooltHeatrActvnCtrl_MemWrForCooltHeatrActvnCtrl_merge/1 -> MemWrForCooltHeatrActvnCtrl_MemWrForCooltHeatrActvnCtrl/1
  MemWrForCooltHeatrCtrl_MemWrForCooltHeatrCtrl_merge/1 -> MemWrForCooltHeatrCtrl_MemWrForCooltHeatrCtrl/1
  MemWrForFuTInFuHeatrMdl_MemWrForFuTInFuHeatrMdl_merge/1 -> MemWrForFuTInFuHeatrMdl_MemWrForFuTInFuHeatrMdl/1
  MemWrForHeatgInpMgr_MemWrForHeatgInpMgr_merge/1 -> MemWrForHeatgInpMgr_MemWrForHeatgInpMgr/1
  MemWrForSnsrMdlCooltT_MemWrForSnsrMdlCooltT_merge/1 -> MemWrForSnsrMdlCooltT_MemWrForSnsrMdlCooltT/1
  SdbRxSigProc_Runnable_sys/284(NetReqForHeatg_NetReqForHeatg_write) -> NetReqForHeatg_NetReqForHeatg/1
  SdbRxSigProc_Runnable_sys/285(OnBdChrgrHndlSts_OnBdChrgrHndlSts_write) -> OnBdChrgrHndlSts_OnBdChrgrHndlSts/1
  SdbRxSigProc_Runnable_sys/286(PassSeatHeatgActlPwrCns_Ngcc_PassSeatHeatgActlPwrCns_write) -> PassSeatHeatgActlPwrCns_Ngcc_PassSeatHeatgActlPwrCns/1
  SdbRxSigProc_Runnable_sys/287(PassSeatHeatgDesPwrCns_Ngcc_PassSeatHeatgDesPwrCns_write) -> PassSeatHeatgDesPwrCns_Ngcc_PassSeatHeatgDesPwrCns/1
  SdbRxSigProc_Runnable_sys/288(PassSeatVentnActlPwrCns_Ngcc_PassSeatVentnActlPwrCns_write) -> PassSeatVentnActlPwrCns_Ngcc_PassSeatVentnActlPwrCns/1
  SdbRxSigProc_Runnable_sys/289(PassSeatVentnDesPwrCns_Ngcc_PassSeatVentnDesPwrCns_write) -> PassSeatVentnDesPwrCns_Ngcc_PassSeatVentnDesPwrCns/1
  SdbRxSigProc_Runnable_sys/290(SplyUForClimaCtrl_SplyUForClimaCtrl_write) -> SplyUForClimaCtrl_SplyUForClimaCtrl/1
  SdbRxSigProc_Runnable_sys/291(PostDrvgClimaReq_Ngcc_PostDrvgClimaReq_write) -> PostDrvgClimaReq_Ngcc_PostDrvgClimaReq/1
  SdbRxSigProc_Runnable_sys/292(PrkgHeatgActv_PrkgHeatgActv_write) -> PrkgHeatgActv_PrkgHeatgActv/1
  SdbRxSigProc_Runnable_sys/293(PrkgHeatrStrtd_PrkgHeatrStrtd_write) -> PrkgHeatrStrtd_PrkgHeatrStrtd/1
  SdbRxSigProc_Runnable_sys/294(PwrCnsDesForSeatHeatg_PwrCnsDesForSeatHeatg_write) -> PwrCnsDesForSeatHeatg_PwrCnsDesForSeatHeatg/1
  SdbRxSigProc_Runnable_sys/295(PwrCnsDesForSeatVent_PwrCnsDesForSeatVent_write) -> PwrCnsDesForSeatVent_PwrCnsDesForSeatVent/1
  SdbRxSigProc_Runnable_sys/296(PwrCnsDurgEngStopForFuHeatr_PwrCnsDurgEngStopForFuHeatr_write) -> PwrCnsDurgEngStopForFuHeatr_PwrCnsDurgEngStopForFuHeatr/1
  SdbRxSigProc_Runnable_sys/297(PwrCnsDurgEngStopForHvacCooltVlv_PwrCnsDurgEngStopForHvacCooltVlv_write) -> PwrCnsDurgEngStopForHvacCooltVlv_PwrCnsDurgEngStopForHvacCooltVlv/1
  SdbRxSigProc_Runnable_sys/298(PwrCnsDurgEngStopForWtrPmpAux_PwrCnsDurgEngStopForWtrPmpAux_write) -> PwrCnsDurgEngStopForWtrPmpAux_PwrCnsDurgEngStopForWtrPmpAux/1
  SdbRxSigProc_Runnable_sys/299(PwrCnsForFuHeatr_PwrCnsForFuHeatr_write) -> PwrCnsForFuHeatr_PwrCnsForFuHeatr/1
  SdbRxSigProc_Runnable_sys/300(PwrCnsForHvacCooltVlv_PwrCnsForHvacCooltVlv_write) -> PwrCnsForHvacCooltVlv_PwrCnsForHvacCooltVlv/1
  SdbRxSigProc_Runnable_sys/301(PwrCnsForHvacHeatrElecFrnt_PwrCnsForHvacHeatrElecFrnt_write) -> PwrCnsForHvacHeatrElecFrnt_PwrCnsForHvacHeatrElecFrnt/1
  SdbRxSigProc_Runnable_sys/302(PwrCnsForSeatHeatg_PwrCnsForSeatHeatg_write) -> PwrCnsForSeatHeatg_PwrCnsForSeatHeatg/1
  SdbRxSigProc_Runnable_sys/303(PwrCnsForWtrPmpAux_PwrCnsForWtrPmpAux_write) -> PwrCnsForWtrPmpAux_PwrCnsForWtrPmpAux/1
  SdbRxSigProc_Runnable_sys/304(PwrStsForPrkgHeatrElec_PwrStsForPrkgHeatrElec_write) -> PwrStsForPrkgHeatrElec_PwrStsForPrkgHeatrElec/1
  SdbRxSigProc_Runnable_sys/305(RainfallAmnt_Ngcc_RainfallAmnt_write) -> RainfallAmnt_Ngcc_RainfallAmnt/1
  SdbRxSigProc_Runnable_sys/306(RemStrtHvCtrlReq_Ngcc_RemStrtHvCtrlReq_write) -> RemStrtHvCtrlReq_Ngcc_RemStrtHvCtrlReq/1
  SdbRxSigProc_Runnable_sys/307(SeatHeatDurgClimaEnad_SeatHeatDurgClimaEnad_write) -> SeatHeatDurgClimaEnad_SeatHeatDurgClimaEnad/1
  SdbRxSigProc_Runnable_sys/308(SeatHeatDurgClimaEnadConnGen2_SeatHeatDurgClimaEnadConnGen2_write) -> SeatHeatDurgClimaEnadConnGen2_SeatHeatDurgClimaEnadConnGen2/1
  SdbRxSigProc_Runnable_sys/309(SeatHeatDurgClimaEnadFromTelm_Ngcc_SeatHeatDurgClimaEnadFromTelm_write) -> SeatHeatDurgClimaEnadFromTelm_Ngcc_SeatHeatDurgClimaEnadFromTelm/1
  SdbRxSigProc_Runnable_sys/310(SecRowSeatHeatgActlPwrCnsLe_Ngcc_SecRowSeatHeatgActlPwrCnsLe_write) -> SecRowSeatHeatgActlPwrCnsLe_Ngcc_SecRowSeatHeatgActlPwrCnsLe/1
  SdbRxSigProc_Runnable_sys/311(SecRowSeatHeatgActlPwrCnsRi_Ngcc_SecRowSeatHeatgActlPwrCnsRi_write) -> SecRowSeatHeatgActlPwrCnsRi_Ngcc_SecRowSeatHeatgActlPwrCnsRi/1
  SdbRxSigProc_Runnable_sys/312(SecRowSeatHeatgDesPwrCnsLe_Ngcc_SecRowSeatHeatgDesPwrCnsLe_write) -> SecRowSeatHeatgDesPwrCnsLe_Ngcc_SecRowSeatHeatgDesPwrCnsLe/1
  SdbRxSigProc_Runnable_sys/313(SecRowSeatHeatgDesPwrCnsRi_Ngcc_SecRowSeatHeatgDesPwrCnsRi_write) -> SecRowSeatHeatgDesPwrCnsRi_Ngcc_SecRowSeatHeatgDesPwrCnsRi/1
  SdbRxSigProc_Runnable_sys/314(SecRowSeatVentnActlPwrCnsLe_Ngcc_SecRowSeatVentnActlPwrCnsLe_write) -> SecRowSeatVentnActlPwrCnsLe_Ngcc_SecRowSeatVentnActlPwrCnsLe/1
  SdbRxSigProc_Runnable_sys/315(SecRowSeatVentnActlPwrCnsRi_Ngcc_SecRowSeatVentnActlPwrCnsRi_write) -> SecRowSeatVentnActlPwrCnsRi_Ngcc_SecRowSeatVentnActlPwrCnsRi/1
  SdbRxSigProc_Runnable_sys/316(SecRowSeatVentnDesPwrCnsLe_Ngcc_SecRowSeatVentnDesPwrCnsLe_write) -> SecRowSeatVentnDesPwrCnsLe_Ngcc_SecRowSeatVentnDesPwrCnsLe/1
  SdbRxSigProc_Runnable_sys/317(SecRowSeatVentnDesPwrCnsRi_Ngcc_SecRowSeatVentnDesPwrCnsRi_write) -> SecRowSeatVentnDesPwrCnsRi_Ngcc_SecRowSeatVentnDesPwrCnsRi/1
  SdbRxSigProc_Runnable_sys/318(SessionActvOutOfVeh_SessionActvOutOfVeh_write) -> SessionActvOutOfVeh_SessionActvOutOfVeh/1
  SdbRxSigProc_Runnable_sys/319(SteerWhlHeatgDesPwr_Ngcc_SteerWhlHeatgDesPwr_write) -> SteerWhlHeatgDesPwr_Ngcc_SteerWhlHeatgDesPwr/1
  SdbRxSigProc_Runnable_sys/320(SteerWhlHeatgDurgClimaEnad_SteerWhlHeatgDurgClimaEnad_write) -> SteerWhlHeatgDurgClimaEnad_SteerWhlHeatgDurgClimaEnad/1
  SdbRxSigProc_Runnable_sys/321(SteerWhlHeatgDurgClimEandFromTelmConnGen2_SteerWhlHeatgDurgClimEandFromTelmConnGen2_write) -> SteerWhlHeatgDurgClimEandFromTelmConnGen2_SteerWhlHeatgDurgClimEandFromTelmConnGen2/1
  SdbRxSigProc_Runnable_sys/322(SteerWhlHeatgPwrAct_Ngcc_SteerWhlHeatgPwrAct_write) -> SteerWhlHeatgPwrAct_Ngcc_SteerWhlHeatgPwrAct/1
  SdbRxSigProc_Runnable_sys/323(StrtRemSts_StrtRemSts1WdStrtRemSts_write) -> StrtRemSts_StrtRemSts1WdStrtRemSts/1
  SdbRxSigProc_Runnable_sys/324(StrtRemSts1WdStrtRemSts_Ngcc_StrtRemSts1WdStrtRemSts_write) -> StrtRemSts1WdStrtRemSts_Ngcc_StrtRemSts1WdStrtRemSts/1
  SdbRxSigProc_Runnable_sys/325(SunCurtPosnSts_Ngcc_SunCurtPosnSts_write) -> SunCurtPosnSts_Ngcc_SunCurtPosnSts/1
  SunData_SunData_merge/1 -> SunData_SunData/1
  SunLoadOutOfVeh_SunLoadOutOfVeh_merge/1 -> SunLoadOutOfVeh_SunLoadOutOfVeh/1
  SdbRxSigProc_Runnable_sys/328(SunRoofPosnSts_Ngcc_SunRoofPosnSts_write) -> SunRoofPosnSts_Ngcc_SunRoofPosnSts/1
  SdbRxSigProc_Runnable_sys/329(TelmPM25Req_Ngcc_TelmPM25Req_write) -> TelmPM25Req_Ngcc_TelmPM25Req/1
  SdbRxSigProc_Runnable_sys/330(TiDrvgCycPred_TiDrvgCycPred_write) -> TiDrvgCycPred_TiDrvgCycPred/1
  SdbRxSigProc_Runnable_sys/331(TrSts_Ngcc_TrSts_write) -> TrSts_Ngcc_TrSts/1
  SdbRxSigProc_Runnable_sys/332(VehModMngtGlbSafe1_Ngcc_VehModMngtGlbSafe1_write) -> VehModMngtGlbSafe1_Ngcc_VehModMngtGlbSafe1/1
  SdbRxSigProc_Runnable_sys/333(VehSpdLgt_Ngcc_VehSpdLgtSafe_write) -> VehSpdLgt_Ngcc_VehSpdLgtSafe/1
  SdbRxSigProc_Runnable_sys/334(WadeProtnSts_Ngcc_WadeProtnSts_write) -> WadeProtnSts_Ngcc_WadeProtnSts/1
  SdbRxSigProc_Runnable_sys/335(WinGlbCmd_Ngcc_WinGlbCmd_write) -> WinGlbCmd_Ngcc_WinGlbCmd/1
  SdbRxSigProc_Runnable_sys/336(WinPosnStsAtDrvrReNgcc_WinPosnStsAtDrvrReNgcc_write) -> WinPosnStsAtDrvrReNgcc_WinPosnStsAtDrvrReNgcc/1
  SdbRxSigProc_Runnable_sys/337(WinPosnStsAtPassReNgcc_WinPosnStsAtPassRe_write) -> WinPosnStsAtPassReNgcc_WinPosnStsAtPassRe/1
  SdbRxSigProc_Runnable_sys/338(WinPosnStsPass_WinPosnStsAtPass_write) -> WinPosnStsPass_WinPosnStsAtPass/1
  SdbRxSigProc_Runnable_sys/339(WipgInfo_Ngcc_WipgInfo_write) -> WipgInfo_Ngcc_WipgInfo/1
  SdbRxSigProc_Runnable_sys/340(WtrPmpActvnReq_WtrPmpActvnReq_write) -> WtrPmpActvnReq_WtrPmpActvnReq/1
  SdbRxSigProc_Runnable_sys/327(SunLoadOutOfVeh_SunLoadOutOfVeh_write) -> [SunLoadOutOfVeh_SunLoadOutOfVeh_merge_Tag]
  [SunLoadOutOfVeh_SunLoadOutOfVeh_merge_Tag] -> SunLoadOutOfVeh_SunLoadOutOfVeh_merge/2
  SdbRxSigProc_Init/16(SunLoadOutOfVeh_SunLoadOutOfVeh_write) -> [SunLoadOutOfVeh_SunLoadOutOfVeh_merge_Tag1]
  [SunLoadOutOfVeh_SunLoadOutOfVeh_merge_Tag1] -> SunLoadOutOfVeh_SunLoadOutOfVeh_merge/1
  SdbRxSigProc_Runnable_sys/326(SunData_SunData_write) -> [SunData_SunData_merge_Tag]
  [SunData_SunData_merge_Tag] -> SunData_SunData_merge/2
  SdbRxSigProc_Init/15(SunData_SunData_write) -> [SunData_SunData_merge_Tag1]
  [SunData_SunData_merge_Tag1] -> SunData_SunData_merge/1
  SdbRxSigProc_Runnable_sys/283(MemWrForSnsrMdlCooltT_MemWrForSnsrMdlCooltT_write) -> [MemWrForSnsrMdlCooltT_MemWrForSnsrMdlCooltT_merge_Tag]
  [MemWrForSnsrMdlCooltT_MemWrForSnsrMdlCooltT_merge_Tag] -> MemWrForSnsrMdlCooltT_MemWrForSnsrMdlCooltT_merge/2
  SdbRxSigProc_Init/14(MemWrForSnsrMdlCooltT_MemWrForSnsrMdlCooltT_write) -> [MemWrForSnsrMdlCooltT_MemWrForSnsrMdlCooltT_merge_Tag1]
  [MemWrForSnsrMdlCooltT_MemWrForSnsrMdlCooltT_merge_Tag1] -> MemWrForSnsrMdlCooltT_MemWrForSnsrMdlCooltT_merge/1
  SdbRxSigProc_Runnable_sys/282(MemWrForHeatgInpMgr_MemWrForHeatgInpMgr_write) -> [MemWrForHeatgInpMgr_MemWrForHeatgInpMgr_merge_Tag]
  [MemWrForHeatgInpMgr_MemWrForHeatgInpMgr_merge_Tag] -> MemWrForHeatgInpMgr_MemWrForHeatgInpMgr_merge/2
  SdbRxSigProc_Init/13(MemWrForHeatgInpMgr_MemWrForHeatgInpMgr_write) -> [MemWrForHeatgInpMgr_MemWrForHeatgInpMgr_merge_Tag1]
  [MemWrForHeatgInpMgr_MemWrForHeatgInpMgr_merge_Tag1] -> MemWrForHeatgInpMgr_MemWrForHeatgInpMgr_merge/1
  SdbRxSigProc_Runnable_sys/281(MemWrForFuTInFuHeatrMdl_MemWrForFuTInFuHeatrMdl_write) -> [MemWrForFuTInFuHeatrMdl_MemWrForFuTInFuHeatrMdl_merge_Tag]
  [MemWrForFuTInFuHeatrMdl_MemWrForFuTInFuHeatrMdl_merge_Tag] -> MemWrForFuTInFuHeatrMdl_MemWrForFuTInFuHeatrMdl_merge/2
  SdbRxSigProc_Init/12(MemWrForFuTInFuHeatrMdl_MemWrForFuTInFuHeatrMdl_write) -> [MemWrForFuTInFuHeatrMdl_MemWrForFuTInFuHeatrMdl_merge_Tag1]
  [MemWrForFuTInFuHeatrMdl_MemWrForFuTInFuHeatrMdl_merge_Tag1] -> MemWrForFuTInFuHeatrMdl_MemWrForFuTInFuHeatrMdl_merge/1
  SdbRxSigProc_Runnable_sys/280(MemWrForCooltHeatrCtrl_MemWrForCooltHeatrCtrl_write) -> [MemWrForCooltHeatrCtrl_MemWrForCooltHeatrCtrl_merge_Tag]
  [MemWrForCooltHeatrCtrl_MemWrForCooltHeatrCtrl_merge_Tag] -> MemWrForCooltHeatrCtrl_MemWrForCooltHeatrCtrl_merge/2
  SdbRxSigProc_Init/11(MemWrForCooltHeatrCtrl_MemWrForCooltHeatrCtrl_write) -> [MemWrForCooltHeatrCtrl_MemWrForCooltHeatrCtrl_merge_Tag1]
  [MemWrForCooltHeatrCtrl_MemWrForCooltHeatrCtrl_merge_Tag1] -> MemWrForCooltHeatrCtrl_MemWrForCooltHeatrCtrl_merge/1
  SdbRxSigProc_Runnable_sys/279(MemWrForCooltHeatrActvnCtrl_MemWrForCooltHeatrActvnCtrl_write) -> [MemWrForCooltHeatrActvnCtrl_MemWrForCooltHeatr_69f7fed298b7276a]
  [MemWrForCooltHeatrActvnCtrl_MemWrForCooltHeatr_69f7fed298b7276a] -> MemWrForCooltHeatrActvnCtrl_MemWrForCooltHeatrActvnCtrl_merge/2
  SdbRxSigProc_Init/10(MemWrForCooltHeatrActvnCtrl_MemWrForCooltHeatrActvnCtrl_write) -> [MemWrForCooltHeatrActvnCtrl_MemWrForCooltHeatr_6194abaa96ef69e6]
  [MemWrForCooltHeatrActvnCtrl_MemWrForCooltHeatr_6194abaa96ef69e6] -> MemWrForCooltHeatrActvnCtrl_MemWrForCooltHeatrActvnCtrl_merge/1
  SdbRxSigProc_Runnable_sys/271(IsgMotTInt_IsgMotTInt_write) -> [IsgMotTInt_IsgMotTInt_merge_Tag]
  [IsgMotTInt_IsgMotTInt_merge_Tag] -> IsgMotTInt_IsgMotTInt_merge/2
  SdbRxSigProc_Init/9(IsgMotTInt_IsgMotTInt_write) -> [IsgMotTInt_IsgMotTInt_merge_Tag1]
  [IsgMotTInt_IsgMotTInt_merge_Tag1] -> IsgMotTInt_IsgMotTInt_merge/1
  SdbRxSigProc_Runnable_sys/263(HvBattCoolgPwrHeatgMgrThermPred_HvBattCoolgPwrHeatgMgrThermPred_write) -> [HvBattCoolgPwrHeatgMgrThermPred_HvBattCoolgPwr_d28efc3b938bd68f]
  [HvBattCoolgPwrHeatgMgrThermPred_HvBattCoolgPwr_d28efc3b938bd68f] -> HvBattCoolgPwrHeatgMgrThermPred_HvBattCoolgPwrHeatgMgrThermPred_merge/2
  SdbRxSigProc_Init/8(HvBattCoolgPwrHeatgMgrThermPred_HvBattCoolgPwrHeatgMgrThermPred_write) -> [HvBattCoolgPwrHeatgMgrThermPred_HvBattCoolgPwr_fa3d5094d8830506]
  [HvBattCoolgPwrHeatgMgrThermPred_HvBattCoolgPwr_fa3d5094d8830506] -> HvBattCoolgPwrHeatgMgrThermPred_HvBattCoolgPwrHeatgMgrThermPred_merge/1
  SdbRxSigProc_Runnable_sys/258(HumOutOfVeh_HumOutOfVeh_write) -> [HumOutOfVeh_HumOutOfVeh_merge_Tag]
  [HumOutOfVeh_HumOutOfVeh_merge_Tag] -> HumOutOfVeh_HumOutOfVeh_merge/2
  SdbRxSigProc_Init/7(HumOutOfVeh_HumOutOfVeh_write) -> [HumOutOfVeh_HumOutOfVeh_merge_Tag1]
  [HumOutOfVeh_HumOutOfVeh_merge_Tag1] -> HumOutOfVeh_HumOutOfVeh_merge/1
  SdbRxSigProc_Runnable_sys/242(FogOutOfVeh_FogOutOfVeh_write) -> [FogOutOfVeh_FogOutOfVeh_merge_Tag]
  [FogOutOfVeh_FogOutOfVeh_merge_Tag] -> FogOutOfVeh_FogOutOfVeh_merge/2
  SdbRxSigProc_Init/6(FogOutOfVeh_FogOutOfVeh_write) -> [FogOutOfVeh_FogOutOfVeh_merge_Tag1]
  [FogOutOfVeh_FogOutOfVeh_merge_Tag1] -> FogOutOfVeh_FogOutOfVeh_merge/1
  SdbRxSigProc_Runnable_sys/241(EngT_Ngcc_EngT_write) -> [EngT_Ngcc_EngT_merge_Tag]
  [EngT_Ngcc_EngT_merge_Tag] -> EngT_Ngcc_EngT_merge/2
  SdbRxSigProc_Init/5(EngT_Ngcc_EngT_write) -> [EngT_Ngcc_EngT_merge_Tag1]
  [EngT_Ngcc_EngT_merge_Tag1] -> EngT_Ngcc_EngT_merge/1
  SdbRxSigProc_Runnable_sys/221(CooltHeatrAvlStsForPrkgClima_CooltHeatrAvlStsForPrkgClima_write) -> [CooltHeatrAvlStsForPrkgClima_CooltHeatrAvlStsF_26ab44e24cdb1984]
  [CooltHeatrAvlStsForPrkgClima_CooltHeatrAvlStsF_26ab44e24cdb1984] -> CooltHeatrAvlStsForPrkgClima_CooltHeatrAvlStsForPrkgClima_merge/2
  SdbRxSigProc_Init/4(CooltHeatrAvlStsForPrkgClima_CooltHeatrAvlStsForPrkgClima_write) -> [CooltHeatrAvlStsForPrkgClima_CooltHeatrAvlStsF_9e7afe918af83492]
  [CooltHeatrAvlStsForPrkgClima_CooltHeatrAvlStsF_9e7afe918af83492] -> CooltHeatrAvlStsForPrkgClima_CooltHeatrAvlStsForPrkgClima_merge/1
  SdbRxSigProc_Runnable_sys/217(CmptmtRelHum_Ngcc_CmptmtRelHum_write) -> [CmptmtRelHum_Ngcc_CmptmtRelHum_merge_Tag]
  [CmptmtRelHum_Ngcc_CmptmtRelHum_merge_Tag] -> CmptmtRelHum_Ngcc_CmptmtRelHum_merge/2
  SdbRxSigProc_Init/3(CmptmtRelHum_Ngcc_CmptmtRelHum_write) -> [CmptmtRelHum_Ngcc_CmptmtRelHum_merge_Tag1]
  [CmptmtRelHum_Ngcc_CmptmtRelHum_merge_Tag1] -> CmptmtRelHum_Ngcc_CmptmtRelHum_merge/1
  SdbRxSigProc_Runnable_sys/193(AmbTRaw_Ngcc_AmbTRaw_write) -> [AmbTRaw_Ngcc_AmbTRaw_merge_Tag]
  [AmbTRaw_Ngcc_AmbTRaw_merge_Tag] -> AmbTRaw_Ngcc_AmbTRaw_merge/2
  SdbRxSigProc_Init/2(AmbTRaw_Ngcc_AmbTRaw_write) -> [AmbTRaw_Ngcc_AmbTRaw_merge_Tag1]
  [AmbTRaw_Ngcc_AmbTRaw_merge_Tag1] -> AmbTRaw_Ngcc_AmbTRaw_merge/1
  SdbRxSigProc_Runnable_sys/192(AmbTOutOfVeh_AmbTOutOfVeh_write) -> [AmbTOutOfVeh_AmbTOutOfVeh_merge_Tag]
  [AmbTOutOfVeh_AmbTOutOfVeh_merge_Tag] -> AmbTOutOfVeh_AmbTOutOfVeh_merge/2
  SdbRxSigProc_Init/1(AmbTOutOfVeh_AmbTOutOfVeh_write) -> [AmbTOutOfVeh_AmbTOutOfVeh_merge_Tag1]
  [AmbTOutOfVeh_AmbTOutOfVeh_merge_Tag1] -> AmbTOutOfVeh_AmbTOutOfVeh_merge/1
  SdbRxSigProc_Runnable/1 -> SdbRxSigProc_Runnable_sys/Trigger
